simulink model "l1_ver113"  (Simulink 7.9)
settings: Solver=FixedStepDiscrete  SolverMode=SingleTasking  StartTime=0.0  StopTime=2048  RelTol=1e-3
undefined symbols (not defined in the file): sfp
libraries: xbsIndex_r4 x2413, casper_library_downconverter x256, xps_library x32, dspsigops x32, casper_library_misc x27, casper_library_flow_control x16, simulink x7
(part 5 of 24)

== system l1_ver113/dec_fir3 ==
  sync_in : Inport
  real1 : Inport  Port=2
  imag1 : Inport  Port=3
  real2 : Inport  Port=4
  imag2 : Inport  Port=5
  real3 : Inport  Port=6
  imag3 : Inport  Port=7
  real4 : Inport  Port=8
  imag4 : Inport  Port=9
  real5 : Inport  Port=10
  imag5 : Inport  Port=11
  real6 : Inport  Port=12
  imag6 : Inport  Port=13
  real7 : Inport  Port=14
  imag7 : Inport  Port=15
  real8 : Inport  Port=16
  imag8 : Inport  Port=17
  real9 : Inport  Port=18
  imag9 : Inport  Port=19
  real10 : Inport  Port=20
  imag10 : Inport  Port=21
  real11 : Inport  Port=22
  imag11 : Inport  Port=23
  real12 : Inport  Port=24
  imag12 : Inport  Port=25
  real13 : Inport  Port=26
  imag13 : Inport  Port=27
  real14 : Inport  Port=28
  imag14 : Inport  Port=29
  real15 : Inport  Port=30
  imag15 : Inport  Port=31
  real16 : Inport  Port=32
  imag16 : Inport  Port=33
  convert1 : xbsIndex_r4/Convert  gui_display_data_type=Fixed-point  arith_type="Signed  (2's comp)"  n_bits=32  bin_pt=31  float_type=Single  exp_bits=8  fraction_bits=24  quantization="Round  (unbiased: +/- Inf)"  overflow=Wrap  en=off  latency=2  dbl_ovrd=off  pipeline=off  block_type=convert
  convert2 : xbsIndex_r4/Convert  gui_display_data_type=Fixed-point  arith_type="Signed  (2's comp)"  n_bits=32  bin_pt=31  float_type=Single  exp_bits=8  fraction_bits=24  quantization="Round  (unbiased: +/- Inf)"  overflow=Wrap  en=off  latency=2  dbl_ovrd=off  pipeline=off  block_type=convert
  delay : xbsIndex_r4/Delay  rst=off  infoeditControl=Selection of Reset will increase slice count due to use of real FFs and instead of SRLs  en=off  latency=9  dbl_ovrd=off  reg_retiming=off  block_type=delay
  fir_dbl_col1 : Subsystem  AncestorBlock=casper_library_downconverter/fir_dbl_col  mask(fir_dbl_col){n_inputs=16, coeff=getfield( getfield( get_param( gcb,'UserData' ), 'parameters'),'coeff'), add_latency=1, mult_latency=2, coeff_bit_width=25, coeff_bin_pt=24, first_stage_hdl=on, adder_imp=DSP48}
      mask prompts: n_inputs "Inputs"; coeff "Coefficients"; add_latency "Add latency"; mult_latency "Mult Latency"; coeff_bit_width "Coefficient bit width"; coeff_bin_pt "Coefficient binary point"; first_stage_hdl "Implement first stage of adder trees on output as behavioural HDL"; adder_imp "Adder implementation"
      mask init: same as l1_ver113/dec_fir1/fir_dbl_col1
      mask Help: eval('xlWeb(''http://casper.berkeley.edu/wiki/Fir_dbl_col'')')
      mask StyleString: edit,edit,edit,edit,edit,edit,checkbox,popup(Behavioral|Fabric|DSP48)
  fir_dbl_col2 : Subsystem  AncestorBlock=casper_library_downconverter/fir_dbl_col  mask(fir_dbl_col){n_inputs=16, coeff=getfield( getfield( get_param( gcb,'UserData' ), 'parameters'),'coeff'), add_latency=1, mult_latency=2, coeff_bit_width=25, coeff_bin_pt=24, first_stage_hdl=on, adder_imp=DSP48}
      mask prompts: n_inputs "Inputs"; coeff "Coefficients"; add_latency "Add latency"; mult_latency "Mult Latency"; coeff_bit_width "Coefficient bit width"; coeff_bin_pt "Coefficient binary point"; first_stage_hdl "Implement first stage of adder trees on output as behavioural HDL"; adder_imp "Adder implementation"
      mask init: same as l1_ver113/dec_fir1/fir_dbl_col1
      mask Help: eval('xlWeb(''http://casper.berkeley.edu/wiki/Fir_dbl_col'')')
      mask StyleString: edit,edit,edit,edit,edit,edit,checkbox,popup(Behavioral|Fabric|DSP48)
  fir_dbl_col3 : Subsystem  AncestorBlock=casper_library_downconverter/fir_dbl_col  mask(fir_dbl_col){n_inputs=16, coeff=getfield( getfield( get_param( gcb,'UserData' ), 'parameters'),'coeff'), add_latency=1, mult_latency=2, coeff_bit_width=25, coeff_bin_pt=24, first_stage_hdl=on, adder_imp=DSP48}
      mask prompts: n_inputs "Inputs"; coeff "Coefficients"; add_latency "Add latency"; mult_latency "Mult Latency"; coeff_bit_width "Coefficient bit width"; coeff_bin_pt "Coefficient binary point"; first_stage_hdl "Implement first stage of adder trees on output as behavioural HDL"; adder_imp "Adder implementation"
      mask init: same as l1_ver113/dec_fir1/fir_dbl_col1
      mask Help: eval('xlWeb(''http://casper.berkeley.edu/wiki/Fir_dbl_col'')')
      mask StyleString: edit,edit,edit,edit,edit,edit,checkbox,popup(Behavioral|Fabric|DSP48)
  fir_dbl_col4 : Subsystem  AncestorBlock=casper_library_downconverter/fir_dbl_col  mask(fir_dbl_col){n_inputs=16, coeff=getfield( getfield( get_param( gcb,'UserData' ), 'parameters'),'coeff'), add_latency=1, mult_latency=2, coeff_bit_width=25, coeff_bin_pt=24, first_stage_hdl=on, adder_imp=DSP48}
      mask prompts: n_inputs "Inputs"; coeff "Coefficients"; add_latency "Add latency"; mult_latency "Mult Latency"; coeff_bit_width "Coefficient bit width"; coeff_bin_pt "Coefficient binary point"; first_stage_hdl "Implement first stage of adder trees on output as behavioural HDL"; adder_imp "Adder implementation"
      mask init: same as l1_ver113/dec_fir1/fir_dbl_col1
      mask Help: eval('xlWeb(''http://casper.berkeley.edu/wiki/Fir_dbl_col'')')
      mask StyleString: edit,edit,edit,edit,edit,edit,checkbox,popup(Behavioral|Fabric|DSP48)
  fir_dbl_col5 : Subsystem  AncestorBlock=casper_library_downconverter/fir_dbl_col  mask(fir_dbl_col){n_inputs=16, coeff=getfield( getfield( get_param( gcb,'UserData' ), 'parameters'),'coeff'), add_latency=1, mult_latency=2, coeff_bit_width=25, coeff_bin_pt=24, first_stage_hdl=on, adder_imp=DSP48}
      mask prompts: n_inputs "Inputs"; coeff "Coefficients"; add_latency "Add latency"; mult_latency "Mult Latency"; coeff_bit_width "Coefficient bit width"; coeff_bin_pt "Coefficient binary point"; first_stage_hdl "Implement first stage of adder trees on output as behavioural HDL"; adder_imp "Adder implementation"
      mask init: same as l1_ver113/dec_fir1/fir_dbl_col1
      mask Help: eval('xlWeb(''http://casper.berkeley.edu/wiki/Fir_dbl_col'')')
      mask StyleString: edit,edit,edit,edit,edit,edit,checkbox,popup(Behavioral|Fabric|DSP48)
  fir_dbl_col6 : Subsystem  AncestorBlock=casper_library_downconverter/fir_dbl_col  mask(fir_dbl_col){n_inputs=16, coeff=getfield( getfield( get_param( gcb,'UserData' ), 'parameters'),'coeff'), add_latency=1, mult_latency=2, coeff_bit_width=25, coeff_bin_pt=24, first_stage_hdl=on, adder_imp=DSP48}
      mask prompts: n_inputs "Inputs"; coeff "Coefficients"; add_latency "Add latency"; mult_latency "Mult Latency"; coeff_bit_width "Coefficient bit width"; coeff_bin_pt "Coefficient binary point"; first_stage_hdl "Implement first stage of adder trees on output as behavioural HDL"; adder_imp "Adder implementation"
      mask init: same as l1_ver113/dec_fir1/fir_dbl_col1
      mask Help: eval('xlWeb(''http://casper.berkeley.edu/wiki/Fir_dbl_col'')')
      mask StyleString: edit,edit,edit,edit,edit,edit,checkbox,popup(Behavioral|Fabric|DSP48)
  fir_dbl_col7 : Subsystem  AncestorBlock=casper_library_downconverter/fir_dbl_col  mask(fir_dbl_col){n_inputs=16, coeff=getfield( getfield( get_param( gcb,'UserData' ), 'parameters'),'coeff'), add_latency=1, mult_latency=2, coeff_bit_width=25, coeff_bin_pt=24, first_stage_hdl=on, adder_imp=DSP48}
      mask prompts: n_inputs "Inputs"; coeff "Coefficients"; add_latency "Add latency"; mult_latency "Mult Latency"; coeff_bit_width "Coefficient bit width"; coeff_bin_pt "Coefficient binary point"; first_stage_hdl "Implement first stage of adder trees on output as behavioural HDL"; adder_imp "Adder implementation"
      mask init: same as l1_ver113/dec_fir1/fir_dbl_col1
      mask Help: eval('xlWeb(''http://casper.berkeley.edu/wiki/Fir_dbl_col'')')
      mask StyleString: edit,edit,edit,edit,edit,edit,checkbox,popup(Behavioral|Fabric|DSP48)
  fir_dbl_col8 : Subsystem  AncestorBlock=casper_library_downconverter/fir_dbl_col  mask(fir_dbl_col){n_inputs=16, coeff=getfield( getfield( get_param( gcb,'UserData' ), 'parameters'),'coeff'), add_latency=1, mult_latency=2, coeff_bit_width=25, coeff_bin_pt=24, first_stage_hdl=on, adder_imp=DSP48}
      mask prompts: n_inputs "Inputs"; coeff "Coefficients"; add_latency "Add latency"; mult_latency "Mult Latency"; coeff_bit_width "Coefficient bit width"; coeff_bin_pt "Coefficient binary point"; first_stage_hdl "Implement first stage of adder trees on output as behavioural HDL"; adder_imp "Adder implementation"
      mask init: same as l1_ver113/dec_fir1/fir_dbl_col1
      mask Help: eval('xlWeb(''http://casper.berkeley.edu/wiki/Fir_dbl_col'')')
      mask StyleString: edit,edit,edit,edit,edit,edit,checkbox,popup(Behavioral|Fabric|DSP48)
  imag_sum : Subsystem  AncestorBlock=casper_library_misc/adder_tree  mask(adder_tree){n_inputs=8, latency=1, adder_imp=DSP48, first_stage_hdl=off}
      mask prompts: n_inputs "Number of Inputs"; latency "Add Latency"; adder_imp "Adder implementation"; first_stage_hdl "Use behavioural HDL for first stage of adders"
      mask help:
      | Sums all inputs using a tree of adds and delays.
      |
      | Number of inputs: The number of inputs to the block to be summed
      | Add latency: The number of clock cycles to perform one addition (affects timing)..
      | Adder implementation: choice of fabric or DSP48E core, or behavioral HDL
      | Use behavioural HDL for first stage of adders: Causes the first stage of adders to be absorbed into DSP blocks if multipliers precede them (Virtex 5). Overrides Adder implementation
      mask init:
      | adder_tree_init(gcb, ...
      |     'n_inputs', n_inputs, ...
      |     'latency', latency, ...
      |     'adder_imp', adder_imp, ...
      |     'first_stage_hdl', first_stage_hdl);
      settings: AttributesFormatString=latency 3
      mask Help: eval('xlWeb(''http://casper.berkeley.edu/wiki/Adder_tree'')')
      mask StyleString: edit,edit,popup(Behavioral|Fabric|DSP48),checkbox
  real_sum : Subsystem  AncestorBlock=casper_library_misc/adder_tree  mask(adder_tree){n_inputs=8, latency=1, adder_imp=DSP48, first_stage_hdl=off}
      mask prompts: n_inputs "Number of Inputs"; latency "Add Latency"; adder_imp "Adder implementation"; first_stage_hdl "Use behavioural HDL for first stage of adders"
      mask help:
      | Sums all inputs using a tree of adds and delays.
      |
      | Number of inputs: The number of inputs to the block to be summed
      | Add latency: The number of clock cycles to perform one addition (affects timing)..
      | Adder implementation: choice of fabric or DSP48E core, or behavioral HDL
      | Use behavioural HDL for first stage of adders: Causes the first stage of adders to be absorbed into DSP blocks if multipliers precede them (Virtex 5). Overrides Adder implementation
      mask init:
      | adder_tree_init(gcb, ...
      |     'n_inputs', n_inputs, ...
      |     'latency', latency, ...
      |     'adder_imp', adder_imp, ...
      |     'first_stage_hdl', first_stage_hdl);
      settings: AttributesFormatString=latency 3
      mask Help: eval('xlWeb(''http://casper.berkeley.edu/wiki/Adder_tree'')')
      mask StyleString: edit,edit,popup(Behavioral|Fabric|DSP48),checkbox
  ri_to_c : casper_library_misc/ri_to_c
  shift1 : xbsIndex_r4/Shift  shift_dir=Left  shift_bits=1  en=off  latency=0  precision=Full  arith_type="Signed  (2's comp)"  n_bits=16  bin_pt=14  quantization=Truncate  overflow=Wrap  dbl_ovrd=off  block_type=shift
  shift2 : xbsIndex_r4/Shift  shift_dir=Left  shift_bits=1  en=off  latency=0  precision=Full  arith_type="Signed  (2's comp)"  n_bits=16  bin_pt=14  quantization=Truncate  overflow=Wrap  dbl_ovrd=off  block_type=shift
  sync_out : Outport
  dout : Outport  Port=2
  wires:
  real1/1 -> fir_dbl_col1/1(real1)
  imag1/1 -> fir_dbl_col1/2(imag1)
  real2/1 -> fir_dbl_col1/3(real2)
  imag2/1 -> fir_dbl_col1/4(imag2)
  real3/1 -> fir_dbl_col1/5(real3)
  imag3/1 -> fir_dbl_col1/6(imag3)
  real4/1 -> fir_dbl_col1/7(real4)
  imag4/1 -> fir_dbl_col1/8(imag4)
  real5/1 -> fir_dbl_col1/9(real5)
  imag5/1 -> fir_dbl_col1/10(imag5)
  real6/1 -> fir_dbl_col1/11(real6)
  imag6/1 -> fir_dbl_col1/12(imag6)
  real7/1 -> fir_dbl_col1/13(real7)
  imag7/1 -> fir_dbl_col1/14(imag7)
  real8/1 -> fir_dbl_col1/15(real8)
  imag8/1 -> fir_dbl_col1/16(imag8)
  real9/1 -> fir_dbl_col1/17(real9)
  imag9/1 -> fir_dbl_col1/18(imag9)
  real10/1 -> fir_dbl_col1/19(real10)
  imag10/1 -> fir_dbl_col1/20(imag10)
  real11/1 -> fir_dbl_col1/21(real11)
  imag11/1 -> fir_dbl_col1/22(imag11)
  real12/1 -> fir_dbl_col1/23(real12)
  imag12/1 -> fir_dbl_col1/24(imag12)
  real13/1 -> fir_dbl_col1/25(real13)
  imag13/1 -> fir_dbl_col1/26(imag13)
  real14/1 -> fir_dbl_col1/27(real14)
  imag14/1 -> fir_dbl_col1/28(imag14)
  real15/1 -> fir_dbl_col1/29(real15)
  imag15/1 -> fir_dbl_col1/30(imag15)
  real16/1 -> fir_dbl_col1/31(real16)
  imag16/1 -> fir_dbl_col1/32(imag16)
  fir_dbl_col1/65(real_sum) -> real_sum/2(din1)
  fir_dbl_col1/66(imag_sum) -> imag_sum/2(din1)
  fir_dbl_col1/1(real_out1) -> fir_dbl_col2/1(real1)
  fir_dbl_col1/2(imag_out1) -> fir_dbl_col2/2(imag1)
  fir_dbl_col1/3(real_out2) -> fir_dbl_col2/3(real2)
  fir_dbl_col1/4(imag_out2) -> fir_dbl_col2/4(imag2)
  fir_dbl_col1/5(real_out3) -> fir_dbl_col2/5(real3)
  fir_dbl_col1/6(imag_out3) -> fir_dbl_col2/6(imag3)
  fir_dbl_col1/7(real_out4) -> fir_dbl_col2/7(real4)
  fir_dbl_col1/8(imag_out4) -> fir_dbl_col2/8(imag4)
  fir_dbl_col1/9(real_out5) -> fir_dbl_col2/9(real5)
  fir_dbl_col1/10(imag_out5) -> fir_dbl_col2/10(imag5)
  fir_dbl_col1/11(real_out6) -> fir_dbl_col2/11(real6)
  fir_dbl_col1/12(imag_out6) -> fir_dbl_col2/12(imag6)
  fir_dbl_col1/13(real_out7) -> fir_dbl_col2/13(real7)
  fir_dbl_col1/14(imag_out7) -> fir_dbl_col2/14(imag7)
  fir_dbl_col1/15(real_out8) -> fir_dbl_col2/15(real8)
  fir_dbl_col1/16(imag_out8) -> fir_dbl_col2/16(imag8)
  fir_dbl_col1/17(real_out9) -> fir_dbl_col2/17(real9)
  fir_dbl_col1/18(imag_out9) -> fir_dbl_col2/18(imag9)
  fir_dbl_col1/19(real_out10) -> fir_dbl_col2/19(real10)
  fir_dbl_col1/20(imag_out10) -> fir_dbl_col2/20(imag10)
  fir_dbl_col1/21(real_out11) -> fir_dbl_col2/21(real11)
  fir_dbl_col1/22(imag_out11) -> fir_dbl_col2/22(imag11)
  fir_dbl_col1/23(real_out12) -> fir_dbl_col2/23(real12)
  fir_dbl_col1/24(imag_out12) -> fir_dbl_col2/24(imag12)
  fir_dbl_col1/25(real_out13) -> fir_dbl_col2/25(real13)
  fir_dbl_col1/26(imag_out13) -> fir_dbl_col2/26(imag13)
  fir_dbl_col1/27(real_out14) -> fir_dbl_col2/27(real14)
  fir_dbl_col1/28(imag_out14) -> fir_dbl_col2/28(imag14)
  fir_dbl_col1/29(real_out15) -> fir_dbl_col2/29(real15)
  fir_dbl_col1/30(imag_out15) -> fir_dbl_col2/30(imag15)
  fir_dbl_col1/31(real_out16) -> fir_dbl_col2/31(real16)
  fir_dbl_col1/32(imag_out16) -> fir_dbl_col2/32(imag16)
  fir_dbl_col2/65(real_sum) -> real_sum/3(din2)
  fir_dbl_col2/66(imag_sum) -> imag_sum/3(din2)
  fir_dbl_col2/1(real_out1) -> fir_dbl_col3/1(real1)
  fir_dbl_col2/2(imag_out1) -> fir_dbl_col3/2(imag1)
  fir_dbl_col2/3(real_out2) -> fir_dbl_col3/3(real2)
  fir_dbl_col2/4(imag_out2) -> fir_dbl_col3/4(imag2)
  fir_dbl_col2/5(real_out3) -> fir_dbl_col3/5(real3)
  fir_dbl_col2/6(imag_out3) -> fir_dbl_col3/6(imag3)
  fir_dbl_col2/7(real_out4) -> fir_dbl_col3/7(real4)
  fir_dbl_col2/8(imag_out4) -> fir_dbl_col3/8(imag4)
  fir_dbl_col2/9(real_out5) -> fir_dbl_col3/9(real5)
  fir_dbl_col2/10(imag_out5) -> fir_dbl_col3/10(imag5)
  fir_dbl_col2/11(real_out6) -> fir_dbl_col3/11(real6)
  fir_dbl_col2/12(imag_out6) -> fir_dbl_col3/12(imag6)
  fir_dbl_col2/13(real_out7) -> fir_dbl_col3/13(real7)
  fir_dbl_col2/14(imag_out7) -> fir_dbl_col3/14(imag7)
  fir_dbl_col2/15(real_out8) -> fir_dbl_col3/15(real8)
  fir_dbl_col2/16(imag_out8) -> fir_dbl_col3/16(imag8)
  fir_dbl_col2/17(real_out9) -> fir_dbl_col3/17(real9)
  fir_dbl_col2/18(imag_out9) -> fir_dbl_col3/18(imag9)
  fir_dbl_col2/19(real_out10) -> fir_dbl_col3/19(real10)
  fir_dbl_col2/20(imag_out10) -> fir_dbl_col3/20(imag10)
  fir_dbl_col2/21(real_out11) -> fir_dbl_col3/21(real11)
  fir_dbl_col2/22(imag_out11) -> fir_dbl_col3/22(imag11)
  fir_dbl_col2/23(real_out12) -> fir_dbl_col3/23(real12)
  fir_dbl_col2/24(imag_out12) -> fir_dbl_col3/24(imag12)
  fir_dbl_col2/25(real_out13) -> fir_dbl_col3/25(real13)
  fir_dbl_col2/26(imag_out13) -> fir_dbl_col3/26(imag13)
  fir_dbl_col2/27(real_out14) -> fir_dbl_col3/27(real14)
  fir_dbl_col2/28(imag_out14) -> fir_dbl_col3/28(imag14)
  fir_dbl_col2/29(real_out15) -> fir_dbl_col3/29(real15)
  fir_dbl_col2/30(imag_out15) -> fir_dbl_col3/30(imag15)
  fir_dbl_col2/31(real_out16) -> fir_dbl_col3/31(real16)
  fir_dbl_col2/32(imag_out16) -> fir_dbl_col3/32(imag16)
  fir_dbl_col3/65(real_sum) -> real_sum/4(din3)
  fir_dbl_col3/66(imag_sum) -> imag_sum/4(din3)
  fir_dbl_col3/1(real_out1) -> fir_dbl_col4/1(real1)
  fir_dbl_col3/2(imag_out1) -> fir_dbl_col4/2(imag1)
  fir_dbl_col3/3(real_out2) -> fir_dbl_col4/3(real2)
  fir_dbl_col3/4(imag_out2) -> fir_dbl_col4/4(imag2)
  fir_dbl_col3/5(real_out3) -> fir_dbl_col4/5(real3)
  fir_dbl_col3/6(imag_out3) -> fir_dbl_col4/6(imag3)
  fir_dbl_col3/7(real_out4) -> fir_dbl_col4/7(real4)
  fir_dbl_col3/8(imag_out4) -> fir_dbl_col4/8(imag4)
  fir_dbl_col3/9(real_out5) -> fir_dbl_col4/9(real5)
  fir_dbl_col3/10(imag_out5) -> fir_dbl_col4/10(imag5)
  fir_dbl_col3/11(real_out6) -> fir_dbl_col4/11(real6)
  fir_dbl_col3/12(imag_out6) -> fir_dbl_col4/12(imag6)
  fir_dbl_col3/13(real_out7) -> fir_dbl_col4/13(real7)
  fir_dbl_col3/14(imag_out7) -> fir_dbl_col4/14(imag7)
  fir_dbl_col3/15(real_out8) -> fir_dbl_col4/15(real8)
  fir_dbl_col3/16(imag_out8) -> fir_dbl_col4/16(imag8)
  fir_dbl_col3/17(real_out9) -> fir_dbl_col4/17(real9)
  fir_dbl_col3/18(imag_out9) -> fir_dbl_col4/18(imag9)
  fir_dbl_col3/19(real_out10) -> fir_dbl_col4/19(real10)
  fir_dbl_col3/20(imag_out10) -> fir_dbl_col4/20(imag10)
  fir_dbl_col3/21(real_out11) -> fir_dbl_col4/21(real11)
  fir_dbl_col3/22(imag_out11) -> fir_dbl_col4/22(imag11)
  fir_dbl_col3/23(real_out12) -> fir_dbl_col4/23(real12)
  fir_dbl_col3/24(imag_out12) -> fir_dbl_col4/24(imag12)
  fir_dbl_col3/25(real_out13) -> fir_dbl_col4/25(real13)
  fir_dbl_col3/26(imag_out13) -> fir_dbl_col4/26(imag13)
  fir_dbl_col3/27(real_out14) -> fir_dbl_col4/27(real14)
  fir_dbl_col3/28(imag_out14) -> fir_dbl_col4/28(imag14)
  fir_dbl_col3/29(real_out15) -> fir_dbl_col4/29(real15)
  fir_dbl_col3/30(imag_out15) -> fir_dbl_col4/30(imag15)
  fir_dbl_col3/31(real_out16) -> fir_dbl_col4/31(real16)
  fir_dbl_col3/32(imag_out16) -> fir_dbl_col4/32(imag16)
  fir_dbl_col4/65(real_sum) -> real_sum/5(din4)
  fir_dbl_col4/66(imag_sum) -> imag_sum/5(din4)
  fir_dbl_col4/1(real_out1) -> fir_dbl_col5/1(real1)
  fir_dbl_col4/2(imag_out1) -> fir_dbl_col5/2(imag1)
  fir_dbl_col4/3(real_out2) -> fir_dbl_col5/3(real2)
  fir_dbl_col4/4(imag_out2) -> fir_dbl_col5/4(imag2)
  fir_dbl_col4/5(real_out3) -> fir_dbl_col5/5(real3)
  fir_dbl_col4/6(imag_out3) -> fir_dbl_col5/6(imag3)
  fir_dbl_col4/7(real_out4) -> fir_dbl_col5/7(real4)
  fir_dbl_col4/8(imag_out4) -> fir_dbl_col5/8(imag4)
  fir_dbl_col4/9(real_out5) -> fir_dbl_col5/9(real5)
  fir_dbl_col4/10(imag_out5) -> fir_dbl_col5/10(imag5)
  fir_dbl_col4/11(real_out6) -> fir_dbl_col5/11(real6)
  fir_dbl_col4/12(imag_out6) -> fir_dbl_col5/12(imag6)
  fir_dbl_col4/13(real_out7) -> fir_dbl_col5/13(real7)
  fir_dbl_col4/14(imag_out7) -> fir_dbl_col5/14(imag7)
  fir_dbl_col4/15(real_out8) -> fir_dbl_col5/15(real8)
  fir_dbl_col4/16(imag_out8) -> fir_dbl_col5/16(imag8)
  fir_dbl_col4/17(real_out9) -> fir_dbl_col5/17(real9)
  fir_dbl_col4/18(imag_out9) -> fir_dbl_col5/18(imag9)
  fir_dbl_col4/19(real_out10) -> fir_dbl_col5/19(real10)
  fir_dbl_col4/20(imag_out10) -> fir_dbl_col5/20(imag10)
  fir_dbl_col4/21(real_out11) -> fir_dbl_col5/21(real11)
  fir_dbl_col4/22(imag_out11) -> fir_dbl_col5/22(imag11)
  fir_dbl_col4/23(real_out12) -> fir_dbl_col5/23(real12)
  fir_dbl_col4/24(imag_out12) -> fir_dbl_col5/24(imag12)
  fir_dbl_col4/25(real_out13) -> fir_dbl_col5/25(real13)
  fir_dbl_col4/26(imag_out13) -> fir_dbl_col5/26(imag13)
  fir_dbl_col4/27(real_out14) -> fir_dbl_col5/27(real14)
  fir_dbl_col4/28(imag_out14) -> fir_dbl_col5/28(imag14)
  fir_dbl_col4/29(real_out15) -> fir_dbl_col5/29(real15)
  fir_dbl_col4/30(imag_out15) -> fir_dbl_col5/30(imag15)
  fir_dbl_col4/31(real_out16) -> fir_dbl_col5/31(real16)
  fir_dbl_col4/32(imag_out16) -> fir_dbl_col5/32(imag16)
  fir_dbl_col5/65(real_sum) -> real_sum/6(din5)
  fir_dbl_col5/66(imag_sum) -> imag_sum/6(din5)
  fir_dbl_col5/1(real_out1) -> fir_dbl_col6/1(real1)
  fir_dbl_col5/2(imag_out1) -> fir_dbl_col6/2(imag1)
  fir_dbl_col5/3(real_out2) -> fir_dbl_col6/3(real2)
  fir_dbl_col5/4(imag_out2) -> fir_dbl_col6/4(imag2)
  fir_dbl_col5/5(real_out3) -> fir_dbl_col6/5(real3)
  fir_dbl_col5/6(imag_out3) -> fir_dbl_col6/6(imag3)
  fir_dbl_col5/7(real_out4) -> fir_dbl_col6/7(real4)
  fir_dbl_col5/8(imag_out4) -> fir_dbl_col6/8(imag4)
  fir_dbl_col5/9(real_out5) -> fir_dbl_col6/9(real5)
  fir_dbl_col5/10(imag_out5) -> fir_dbl_col6/10(imag5)
  fir_dbl_col5/11(real_out6) -> fir_dbl_col6/11(real6)
  fir_dbl_col5/12(imag_out6) -> fir_dbl_col6/12(imag6)
  fir_dbl_col5/13(real_out7) -> fir_dbl_col6/13(real7)
  fir_dbl_col5/14(imag_out7) -> fir_dbl_col6/14(imag7)
  fir_dbl_col5/15(real_out8) -> fir_dbl_col6/15(real8)
  fir_dbl_col5/16(imag_out8) -> fir_dbl_col6/16(imag8)
  fir_dbl_col5/17(real_out9) -> fir_dbl_col6/17(real9)
  fir_dbl_col5/18(imag_out9) -> fir_dbl_col6/18(imag9)
  fir_dbl_col5/19(real_out10) -> fir_dbl_col6/19(real10)
  fir_dbl_col5/20(imag_out10) -> fir_dbl_col6/20(imag10)
  fir_dbl_col5/21(real_out11) -> fir_dbl_col6/21(real11)
  fir_dbl_col5/22(imag_out11) -> fir_dbl_col6/22(imag11)
  fir_dbl_col5/23(real_out12) -> fir_dbl_col6/23(real12)
  fir_dbl_col5/24(imag_out12) -> fir_dbl_col6/24(imag12)
  fir_dbl_col5/25(real_out13) -> fir_dbl_col6/25(real13)
  fir_dbl_col5/26(imag_out13) -> fir_dbl_col6/26(imag13)
  fir_dbl_col5/27(real_out14) -> fir_dbl_col6/27(real14)
  fir_dbl_col5/28(imag_out14) -> fir_dbl_col6/28(imag14)
  fir_dbl_col5/29(real_out15) -> fir_dbl_col6/29(real15)
  fir_dbl_col5/30(imag_out15) -> fir_dbl_col6/30(imag15)
  fir_dbl_col5/31(real_out16) -> fir_dbl_col6/31(real16)
  fir_dbl_col5/32(imag_out16) -> fir_dbl_col6/32(imag16)
  fir_dbl_col6/65(real_sum) -> real_sum/7(din6)
  fir_dbl_col6/66(imag_sum) -> imag_sum/7(din6)
  fir_dbl_col6/1(real_out1) -> fir_dbl_col7/1(real1)
  fir_dbl_col6/2(imag_out1) -> fir_dbl_col7/2(imag1)
  fir_dbl_col6/3(real_out2) -> fir_dbl_col7/3(real2)
  fir_dbl_col6/4(imag_out2) -> fir_dbl_col7/4(imag2)
  fir_dbl_col6/5(real_out3) -> fir_dbl_col7/5(real3)
  fir_dbl_col6/6(imag_out3) -> fir_dbl_col7/6(imag3)
  fir_dbl_col6/7(real_out4) -> fir_dbl_col7/7(real4)
  fir_dbl_col6/8(imag_out4) -> fir_dbl_col7/8(imag4)
  fir_dbl_col6/9(real_out5) -> fir_dbl_col7/9(real5)
  fir_dbl_col6/10(imag_out5) -> fir_dbl_col7/10(imag5)
  fir_dbl_col6/11(real_out6) -> fir_dbl_col7/11(real6)
  fir_dbl_col6/12(imag_out6) -> fir_dbl_col7/12(imag6)
  fir_dbl_col6/13(real_out7) -> fir_dbl_col7/13(real7)
  fir_dbl_col6/14(imag_out7) -> fir_dbl_col7/14(imag7)
  fir_dbl_col6/15(real_out8) -> fir_dbl_col7/15(real8)
  fir_dbl_col6/16(imag_out8) -> fir_dbl_col7/16(imag8)
  fir_dbl_col6/17(real_out9) -> fir_dbl_col7/17(real9)
  fir_dbl_col6/18(imag_out9) -> fir_dbl_col7/18(imag9)
  fir_dbl_col6/19(real_out10) -> fir_dbl_col7/19(real10)
  fir_dbl_col6/20(imag_out10) -> fir_dbl_col7/20(imag10)
  fir_dbl_col6/21(real_out11) -> fir_dbl_col7/21(real11)
  fir_dbl_col6/22(imag_out11) -> fir_dbl_col7/22(imag11)
  fir_dbl_col6/23(real_out12) -> fir_dbl_col7/23(real12)
  fir_dbl_col6/24(imag_out12) -> fir_dbl_col7/24(imag12)
  fir_dbl_col6/25(real_out13) -> fir_dbl_col7/25(real13)
  fir_dbl_col6/26(imag_out13) -> fir_dbl_col7/26(imag13)
  fir_dbl_col6/27(real_out14) -> fir_dbl_col7/27(real14)
  fir_dbl_col6/28(imag_out14) -> fir_dbl_col7/28(imag14)
  fir_dbl_col6/29(real_out15) -> fir_dbl_col7/29(real15)
  fir_dbl_col6/30(imag_out15) -> fir_dbl_col7/30(imag15)
  fir_dbl_col6/31(real_out16) -> fir_dbl_col7/31(real16)
  fir_dbl_col6/32(imag_out16) -> fir_dbl_col7/32(imag16)
  fir_dbl_col7/65(real_sum) -> real_sum/8(din7)
  fir_dbl_col7/66(imag_sum) -> imag_sum/8(din7)
  fir_dbl_col7/1(real_out1) -> fir_dbl_col8/1(real1)
  fir_dbl_col7/2(imag_out1) -> fir_dbl_col8/2(imag1)
  fir_dbl_col7/3(real_out2) -> fir_dbl_col8/3(real2)
  fir_dbl_col7/4(imag_out2) -> fir_dbl_col8/4(imag2)
  fir_dbl_col7/5(real_out3) -> fir_dbl_col8/5(real3)
  fir_dbl_col7/6(imag_out3) -> fir_dbl_col8/6(imag3)
  fir_dbl_col7/7(real_out4) -> fir_dbl_col8/7(real4)
  fir_dbl_col7/8(imag_out4) -> fir_dbl_col8/8(imag4)
  fir_dbl_col7/9(real_out5) -> fir_dbl_col8/9(real5)
  fir_dbl_col7/10(imag_out5) -> fir_dbl_col8/10(imag5)
  fir_dbl_col7/11(real_out6) -> fir_dbl_col8/11(real6)
  fir_dbl_col7/12(imag_out6) -> fir_dbl_col8/12(imag6)
  fir_dbl_col7/13(real_out7) -> fir_dbl_col8/13(real7)
  fir_dbl_col7/14(imag_out7) -> fir_dbl_col8/14(imag7)
  fir_dbl_col7/15(real_out8) -> fir_dbl_col8/15(real8)
  fir_dbl_col7/16(imag_out8) -> fir_dbl_col8/16(imag8)
  fir_dbl_col7/17(real_out9) -> fir_dbl_col8/17(real9)
  fir_dbl_col7/18(imag_out9) -> fir_dbl_col8/18(imag9)
  fir_dbl_col7/19(real_out10) -> fir_dbl_col8/19(real10)
  fir_dbl_col7/20(imag_out10) -> fir_dbl_col8/20(imag10)
  fir_dbl_col7/21(real_out11) -> fir_dbl_col8/21(real11)
  fir_dbl_col7/22(imag_out11) -> fir_dbl_col8/22(imag11)
  fir_dbl_col7/23(real_out12) -> fir_dbl_col8/23(real12)
  fir_dbl_col7/24(imag_out12) -> fir_dbl_col8/24(imag12)
  fir_dbl_col7/25(real_out13) -> fir_dbl_col8/25(real13)
  fir_dbl_col7/26(imag_out13) -> fir_dbl_col8/26(imag13)
  fir_dbl_col7/27(real_out14) -> fir_dbl_col8/27(real14)
  fir_dbl_col7/28(imag_out14) -> fir_dbl_col8/28(imag14)
  fir_dbl_col7/29(real_out15) -> fir_dbl_col8/29(real15)
  fir_dbl_col7/30(imag_out15) -> fir_dbl_col8/30(imag15)
  fir_dbl_col7/31(real_out16) -> fir_dbl_col8/31(real16)
  fir_dbl_col7/32(imag_out16) -> fir_dbl_col8/32(imag16)
  fir_dbl_col8/65(real_sum) -> real_sum/9(din8)
  fir_dbl_col8/66(imag_sum) -> imag_sum/9(din8)
  real_sum/2(dout) -> shift1/1
  imag_sum/2(dout) -> shift2/1
  shift1/1 -> convert1/1
  shift2/1 -> convert2/1
  convert1/1 -> ri_to_c/1
  convert2/1 -> ri_to_c/2
  ri_to_c/1 -> dout/1
  sync_in/1 -> delay/1
  delay/1 -> real_sum/1(sync), imag_sum/1(sync)
  real_sum/1(sync_out) -> sync_out/1
  fir_dbl_col2/33(real_back_out1) -> fir_dbl_col1/33(real_back1)
  fir_dbl_col2/34(imag_back_out1) -> fir_dbl_col1/34(imag_back1)
  fir_dbl_col2/35(real_back_out2) -> fir_dbl_col1/35(real_back2)
  fir_dbl_col2/36(imag_back_out2) -> fir_dbl_col1/36(imag_back2)
  fir_dbl_col2/37(real_back_out3) -> fir_dbl_col1/37(real_back3)
  fir_dbl_col2/38(imag_back_out3) -> fir_dbl_col1/38(imag_back3)
  fir_dbl_col2/39(real_back_out4) -> fir_dbl_col1/39(real_back4)
  fir_dbl_col2/40(imag_back_out4) -> fir_dbl_col1/40(imag_back4)
  fir_dbl_col2/41(real_back_out5) -> fir_dbl_col1/41(real_back5)
  fir_dbl_col2/42(imag_back_out5) -> fir_dbl_col1/42(imag_back5)
  fir_dbl_col2/43(real_back_out6) -> fir_dbl_col1/43(real_back6)
  fir_dbl_col2/44(imag_back_out6) -> fir_dbl_col1/44(imag_back6)
  fir_dbl_col2/45(real_back_out7) -> fir_dbl_col1/45(real_back7)
  fir_dbl_col2/46(imag_back_out7) -> fir_dbl_col1/46(imag_back7)
  fir_dbl_col2/47(real_back_out8) -> fir_dbl_col1/47(real_back8)
  fir_dbl_col2/48(imag_back_out8) -> fir_dbl_col1/48(imag_back8)
  fir_dbl_col2/49(real_back_out9) -> fir_dbl_col1/49(real_back9)
  fir_dbl_col2/50(imag_back_out9) -> fir_dbl_col1/50(imag_back9)
  fir_dbl_col2/51(real_back_out10) -> fir_dbl_col1/51(real_back10)
  fir_dbl_col2/52(imag_back_out10) -> fir_dbl_col1/52(imag_back10)
  fir_dbl_col2/53(real_back_out11) -> fir_dbl_col1/53(real_back11)
  fir_dbl_col2/54(imag_back_out11) -> fir_dbl_col1/54(imag_back11)
  fir_dbl_col2/55(real_back_out12) -> fir_dbl_col1/55(real_back12)
  fir_dbl_col2/56(imag_back_out12) -> fir_dbl_col1/56(imag_back12)
  fir_dbl_col2/57(real_back_out13) -> fir_dbl_col1/57(real_back13)
  fir_dbl_col2/58(imag_back_out13) -> fir_dbl_col1/58(imag_back13)
  fir_dbl_col2/59(real_back_out14) -> fir_dbl_col1/59(real_back14)
  fir_dbl_col2/60(imag_back_out14) -> fir_dbl_col1/60(imag_back14)
  fir_dbl_col2/61(real_back_out15) -> fir_dbl_col1/61(real_back15)
  fir_dbl_col2/62(imag_back_out15) -> fir_dbl_col1/62(imag_back15)
  fir_dbl_col2/63(real_back_out16) -> fir_dbl_col1/63(real_back16)
  fir_dbl_col2/64(imag_back_out16) -> fir_dbl_col1/64(imag_back16)
  fir_dbl_col3/33(real_back_out1) -> fir_dbl_col2/33(real_back1)
  fir_dbl_col3/34(imag_back_out1) -> fir_dbl_col2/34(imag_back1)
  fir_dbl_col3/35(real_back_out2) -> fir_dbl_col2/35(real_back2)
  fir_dbl_col3/36(imag_back_out2) -> fir_dbl_col2/36(imag_back2)
  fir_dbl_col3/37(real_back_out3) -> fir_dbl_col2/37(real_back3)
  fir_dbl_col3/38(imag_back_out3) -> fir_dbl_col2/38(imag_back3)
  fir_dbl_col3/39(real_back_out4) -> fir_dbl_col2/39(real_back4)
  fir_dbl_col3/40(imag_back_out4) -> fir_dbl_col2/40(imag_back4)
  fir_dbl_col3/41(real_back_out5) -> fir_dbl_col2/41(real_back5)
  fir_dbl_col3/42(imag_back_out5) -> fir_dbl_col2/42(imag_back5)
  fir_dbl_col3/43(real_back_out6) -> fir_dbl_col2/43(real_back6)
  fir_dbl_col3/44(imag_back_out6) -> fir_dbl_col2/44(imag_back6)
  fir_dbl_col3/45(real_back_out7) -> fir_dbl_col2/45(real_back7)
  fir_dbl_col3/46(imag_back_out7) -> fir_dbl_col2/46(imag_back7)
  fir_dbl_col3/47(real_back_out8) -> fir_dbl_col2/47(real_back8)
  fir_dbl_col3/48(imag_back_out8) -> fir_dbl_col2/48(imag_back8)
  fir_dbl_col3/49(real_back_out9) -> fir_dbl_col2/49(real_back9)
  fir_dbl_col3/50(imag_back_out9) -> fir_dbl_col2/50(imag_back9)
  fir_dbl_col3/51(real_back_out10) -> fir_dbl_col2/51(real_back10)
  fir_dbl_col3/52(imag_back_out10) -> fir_dbl_col2/52(imag_back10)
  fir_dbl_col3/53(real_back_out11) -> fir_dbl_col2/53(real_back11)
  fir_dbl_col3/54(imag_back_out11) -> fir_dbl_col2/54(imag_back11)
  fir_dbl_col3/55(real_back_out12) -> fir_dbl_col2/55(real_back12)
  fir_dbl_col3/56(imag_back_out12) -> fir_dbl_col2/56(imag_back12)
  fir_dbl_col3/57(real_back_out13) -> fir_dbl_col2/57(real_back13)
  fir_dbl_col3/58(imag_back_out13) -> fir_dbl_col2/58(imag_back13)
  fir_dbl_col3/59(real_back_out14) -> fir_dbl_col2/59(real_back14)
  fir_dbl_col3/60(imag_back_out14) -> fir_dbl_col2/60(imag_back14)
  fir_dbl_col3/61(real_back_out15) -> fir_dbl_col2/61(real_back15)
  fir_dbl_col3/62(imag_back_out15) -> fir_dbl_col2/62(imag_back15)
  fir_dbl_col3/63(real_back_out16) -> fir_dbl_col2/63(real_back16)
  fir_dbl_col3/64(imag_back_out16) -> fir_dbl_col2/64(imag_back16)
  fir_dbl_col4/33(real_back_out1) -> fir_dbl_col3/33(real_back1)
  fir_dbl_col4/34(imag_back_out1) -> fir_dbl_col3/34(imag_back1)
  fir_dbl_col4/35(real_back_out2) -> fir_dbl_col3/35(real_back2)
  fir_dbl_col4/36(imag_back_out2) -> fir_dbl_col3/36(imag_back2)
  fir_dbl_col4/37(real_back_out3) -> fir_dbl_col3/37(real_back3)
  fir_dbl_col4/38(imag_back_out3) -> fir_dbl_col3/38(imag_back3)
  fir_dbl_col4/39(real_back_out4) -> fir_dbl_col3/39(real_back4)
  fir_dbl_col4/40(imag_back_out4) -> fir_dbl_col3/40(imag_back4)
  fir_dbl_col4/41(real_back_out5) -> fir_dbl_col3/41(real_back5)
  fir_dbl_col4/42(imag_back_out5) -> fir_dbl_col3/42(imag_back5)
  fir_dbl_col4/43(real_back_out6) -> fir_dbl_col3/43(real_back6)
  fir_dbl_col4/44(imag_back_out6) -> fir_dbl_col3/44(imag_back6)
  fir_dbl_col4/45(real_back_out7) -> fir_dbl_col3/45(real_back7)
  fir_dbl_col4/46(imag_back_out7) -> fir_dbl_col3/46(imag_back7)
  fir_dbl_col4/47(real_back_out8) -> fir_dbl_col3/47(real_back8)
  fir_dbl_col4/48(imag_back_out8) -> fir_dbl_col3/48(imag_back8)
  fir_dbl_col4/49(real_back_out9) -> fir_dbl_col3/49(real_back9)
  fir_dbl_col4/50(imag_back_out9) -> fir_dbl_col3/50(imag_back9)
  fir_dbl_col4/51(real_back_out10) -> fir_dbl_col3/51(real_back10)
  fir_dbl_col4/52(imag_back_out10) -> fir_dbl_col3/52(imag_back10)
  fir_dbl_col4/53(real_back_out11) -> fir_dbl_col3/53(real_back11)
  fir_dbl_col4/54(imag_back_out11) -> fir_dbl_col3/54(imag_back11)
  fir_dbl_col4/55(real_back_out12) -> fir_dbl_col3/55(real_back12)
  fir_dbl_col4/56(imag_back_out12) -> fir_dbl_col3/56(imag_back12)
  fir_dbl_col4/57(real_back_out13) -> fir_dbl_col3/57(real_back13)
  fir_dbl_col4/58(imag_back_out13) -> fir_dbl_col3/58(imag_back13)
  fir_dbl_col4/59(real_back_out14) -> fir_dbl_col3/59(real_back14)
  fir_dbl_col4/60(imag_back_out14) -> fir_dbl_col3/60(imag_back14)
  fir_dbl_col4/61(real_back_out15) -> fir_dbl_col3/61(real_back15)
  fir_dbl_col4/62(imag_back_out15) -> fir_dbl_col3/62(imag_back15)
  fir_dbl_col4/63(real_back_out16) -> fir_dbl_col3/63(real_back16)
  fir_dbl_col4/64(imag_back_out16) -> fir_dbl_col3/64(imag_back16)
  fir_dbl_col5/33(real_back_out1) -> fir_dbl_col4/33(real_back1)
  fir_dbl_col5/34(imag_back_out1) -> fir_dbl_col4/34(imag_back1)
  fir_dbl_col5/35(real_back_out2) -> fir_dbl_col4/35(real_back2)
  fir_dbl_col5/36(imag_back_out2) -> fir_dbl_col4/36(imag_back2)
  fir_dbl_col5/37(real_back_out3) -> fir_dbl_col4/37(real_back3)
  fir_dbl_col5/38(imag_back_out3) -> fir_dbl_col4/38(imag_back3)
  fir_dbl_col5/39(real_back_out4) -> fir_dbl_col4/39(real_back4)
  fir_dbl_col5/40(imag_back_out4) -> fir_dbl_col4/40(imag_back4)
  fir_dbl_col5/41(real_back_out5) -> fir_dbl_col4/41(real_back5)
  fir_dbl_col5/42(imag_back_out5) -> fir_dbl_col4/42(imag_back5)
  fir_dbl_col5/43(real_back_out6) -> fir_dbl_col4/43(real_back6)
  fir_dbl_col5/44(imag_back_out6) -> fir_dbl_col4/44(imag_back6)
  fir_dbl_col5/45(real_back_out7) -> fir_dbl_col4/45(real_back7)
  fir_dbl_col5/46(imag_back_out7) -> fir_dbl_col4/46(imag_back7)
  fir_dbl_col5/47(real_back_out8) -> fir_dbl_col4/47(real_back8)
  fir_dbl_col5/48(imag_back_out8) -> fir_dbl_col4/48(imag_back8)
  fir_dbl_col5/49(real_back_out9) -> fir_dbl_col4/49(real_back9)
  fir_dbl_col5/50(imag_back_out9) -> fir_dbl_col4/50(imag_back9)
  fir_dbl_col5/51(real_back_out10) -> fir_dbl_col4/51(real_back10)
  fir_dbl_col5/52(imag_back_out10) -> fir_dbl_col4/52(imag_back10)
  fir_dbl_col5/53(real_back_out11) -> fir_dbl_col4/53(real_back11)
  fir_dbl_col5/54(imag_back_out11) -> fir_dbl_col4/54(imag_back11)
  fir_dbl_col5/55(real_back_out12) -> fir_dbl_col4/55(real_back12)
  fir_dbl_col5/56(imag_back_out12) -> fir_dbl_col4/56(imag_back12)
  fir_dbl_col5/57(real_back_out13) -> fir_dbl_col4/57(real_back13)
  fir_dbl_col5/58(imag_back_out13) -> fir_dbl_col4/58(imag_back13)
  fir_dbl_col5/59(real_back_out14) -> fir_dbl_col4/59(real_back14)
  fir_dbl_col5/60(imag_back_out14) -> fir_dbl_col4/60(imag_back14)
  fir_dbl_col5/61(real_back_out15) -> fir_dbl_col4/61(real_back15)
  fir_dbl_col5/62(imag_back_out15) -> fir_dbl_col4/62(imag_back15)
  fir_dbl_col5/63(real_back_out16) -> fir_dbl_col4/63(real_back16)
  fir_dbl_col5/64(imag_back_out16) -> fir_dbl_col4/64(imag_back16)
  fir_dbl_col6/33(real_back_out1) -> fir_dbl_col5/33(real_back1)
  fir_dbl_col6/34(imag_back_out1) -> fir_dbl_col5/34(imag_back1)
  fir_dbl_col6/35(real_back_out2) -> fir_dbl_col5/35(real_back2)
  fir_dbl_col6/36(imag_back_out2) -> fir_dbl_col5/36(imag_back2)
  fir_dbl_col6/37(real_back_out3) -> fir_dbl_col5/37(real_back3)
  fir_dbl_col6/38(imag_back_out3) -> fir_dbl_col5/38(imag_back3)
  fir_dbl_col6/39(real_back_out4) -> fir_dbl_col5/39(real_back4)
  fir_dbl_col6/40(imag_back_out4) -> fir_dbl_col5/40(imag_back4)
  fir_dbl_col6/41(real_back_out5) -> fir_dbl_col5/41(real_back5)
  fir_dbl_col6/42(imag_back_out5) -> fir_dbl_col5/42(imag_back5)
  fir_dbl_col6/43(real_back_out6) -> fir_dbl_col5/43(real_back6)
  fir_dbl_col6/44(imag_back_out6) -> fir_dbl_col5/44(imag_back6)
  fir_dbl_col6/45(real_back_out7) -> fir_dbl_col5/45(real_back7)
  fir_dbl_col6/46(imag_back_out7) -> fir_dbl_col5/46(imag_back7)
  fir_dbl_col6/47(real_back_out8) -> fir_dbl_col5/47(real_back8)
  fir_dbl_col6/48(imag_back_out8) -> fir_dbl_col5/48(imag_back8)
  fir_dbl_col6/49(real_back_out9) -> fir_dbl_col5/49(real_back9)
  fir_dbl_col6/50(imag_back_out9) -> fir_dbl_col5/50(imag_back9)
  fir_dbl_col6/51(real_back_out10) -> fir_dbl_col5/51(real_back10)
  fir_dbl_col6/52(imag_back_out10) -> fir_dbl_col5/52(imag_back10)
  fir_dbl_col6/53(real_back_out11) -> fir_dbl_col5/53(real_back11)
  fir_dbl_col6/54(imag_back_out11) -> fir_dbl_col5/54(imag_back11)
  fir_dbl_col6/55(real_back_out12) -> fir_dbl_col5/55(real_back12)
  fir_dbl_col6/56(imag_back_out12) -> fir_dbl_col5/56(imag_back12)
  fir_dbl_col6/57(real_back_out13) -> fir_dbl_col5/57(real_back13)
  fir_dbl_col6/58(imag_back_out13) -> fir_dbl_col5/58(imag_back13)
  fir_dbl_col6/59(real_back_out14) -> fir_dbl_col5/59(real_back14)
  fir_dbl_col6/60(imag_back_out14) -> fir_dbl_col5/60(imag_back14)
  fir_dbl_col6/61(real_back_out15) -> fir_dbl_col5/61(real_back15)
  fir_dbl_col6/62(imag_back_out15) -> fir_dbl_col5/62(imag_back15)
  fir_dbl_col6/63(real_back_out16) -> fir_dbl_col5/63(real_back16)
  fir_dbl_col6/64(imag_back_out16) -> fir_dbl_col5/64(imag_back16)
  fir_dbl_col7/33(real_back_out1) -> fir_dbl_col6/33(real_back1)
  fir_dbl_col7/34(imag_back_out1) -> fir_dbl_col6/34(imag_back1)
  fir_dbl_col7/35(real_back_out2) -> fir_dbl_col6/35(real_back2)
  fir_dbl_col7/36(imag_back_out2) -> fir_dbl_col6/36(imag_back2)
  fir_dbl_col7/37(real_back_out3) -> fir_dbl_col6/37(real_back3)
  fir_dbl_col7/38(imag_back_out3) -> fir_dbl_col6/38(imag_back3)
  fir_dbl_col7/39(real_back_out4) -> fir_dbl_col6/39(real_back4)
  fir_dbl_col7/40(imag_back_out4) -> fir_dbl_col6/40(imag_back4)
  fir_dbl_col7/41(real_back_out5) -> fir_dbl_col6/41(real_back5)
  fir_dbl_col7/42(imag_back_out5) -> fir_dbl_col6/42(imag_back5)
  fir_dbl_col7/43(real_back_out6) -> fir_dbl_col6/43(real_back6)
  fir_dbl_col7/44(imag_back_out6) -> fir_dbl_col6/44(imag_back6)
  fir_dbl_col7/45(real_back_out7) -> fir_dbl_col6/45(real_back7)
  fir_dbl_col7/46(imag_back_out7) -> fir_dbl_col6/46(imag_back7)
  fir_dbl_col7/47(real_back_out8) -> fir_dbl_col6/47(real_back8)
  fir_dbl_col7/48(imag_back_out8) -> fir_dbl_col6/48(imag_back8)
  fir_dbl_col7/49(real_back_out9) -> fir_dbl_col6/49(real_back9)
  fir_dbl_col7/50(imag_back_out9) -> fir_dbl_col6/50(imag_back9)
  fir_dbl_col7/51(real_back_out10) -> fir_dbl_col6/51(real_back10)
  fir_dbl_col7/52(imag_back_out10) -> fir_dbl_col6/52(imag_back10)
  fir_dbl_col7/53(real_back_out11) -> fir_dbl_col6/53(real_back11)
  fir_dbl_col7/54(imag_back_out11) -> fir_dbl_col6/54(imag_back11)
  fir_dbl_col7/55(real_back_out12) -> fir_dbl_col6/55(real_back12)
  fir_dbl_col7/56(imag_back_out12) -> fir_dbl_col6/56(imag_back12)
  fir_dbl_col7/57(real_back_out13) -> fir_dbl_col6/57(real_back13)
  fir_dbl_col7/58(imag_back_out13) -> fir_dbl_col6/58(imag_back13)
  fir_dbl_col7/59(real_back_out14) -> fir_dbl_col6/59(real_back14)
  fir_dbl_col7/60(imag_back_out14) -> fir_dbl_col6/60(imag_back14)
  fir_dbl_col7/61(real_back_out15) -> fir_dbl_col6/61(real_back15)
  fir_dbl_col7/62(imag_back_out15) -> fir_dbl_col6/62(imag_back15)
  fir_dbl_col7/63(real_back_out16) -> fir_dbl_col6/63(real_back16)
  fir_dbl_col7/64(imag_back_out16) -> fir_dbl_col6/64(imag_back16)
  fir_dbl_col8/33(real_back_out1) -> fir_dbl_col7/33(real_back1)
  fir_dbl_col8/34(imag_back_out1) -> fir_dbl_col7/34(imag_back1)
  fir_dbl_col8/35(real_back_out2) -> fir_dbl_col7/35(real_back2)
  fir_dbl_col8/36(imag_back_out2) -> fir_dbl_col7/36(imag_back2)
  fir_dbl_col8/37(real_back_out3) -> fir_dbl_col7/37(real_back3)
  fir_dbl_col8/38(imag_back_out3) -> fir_dbl_col7/38(imag_back3)
  fir_dbl_col8/39(real_back_out4) -> fir_dbl_col7/39(real_back4)
  fir_dbl_col8/40(imag_back_out4) -> fir_dbl_col7/40(imag_back4)
  fir_dbl_col8/41(real_back_out5) -> fir_dbl_col7/41(real_back5)
  fir_dbl_col8/42(imag_back_out5) -> fir_dbl_col7/42(imag_back5)
  fir_dbl_col8/43(real_back_out6) -> fir_dbl_col7/43(real_back6)
  fir_dbl_col8/44(imag_back_out6) -> fir_dbl_col7/44(imag_back6)
  fir_dbl_col8/45(real_back_out7) -> fir_dbl_col7/45(real_back7)
  fir_dbl_col8/46(imag_back_out7) -> fir_dbl_col7/46(imag_back7)
  fir_dbl_col8/47(real_back_out8) -> fir_dbl_col7/47(real_back8)
  fir_dbl_col8/48(imag_back_out8) -> fir_dbl_col7/48(imag_back8)
  fir_dbl_col8/49(real_back_out9) -> fir_dbl_col7/49(real_back9)
  fir_dbl_col8/50(imag_back_out9) -> fir_dbl_col7/50(imag_back9)
  fir_dbl_col8/51(real_back_out10) -> fir_dbl_col7/51(real_back10)
  fir_dbl_col8/52(imag_back_out10) -> fir_dbl_col7/52(imag_back10)
  fir_dbl_col8/53(real_back_out11) -> fir_dbl_col7/53(real_back11)
  fir_dbl_col8/54(imag_back_out11) -> fir_dbl_col7/54(imag_back11)
  fir_dbl_col8/55(real_back_out12) -> fir_dbl_col7/55(real_back12)
  fir_dbl_col8/56(imag_back_out12) -> fir_dbl_col7/56(imag_back12)
  fir_dbl_col8/57(real_back_out13) -> fir_dbl_col7/57(real_back13)
  fir_dbl_col8/58(imag_back_out13) -> fir_dbl_col7/58(imag_back13)
  fir_dbl_col8/59(real_back_out14) -> fir_dbl_col7/59(real_back14)
  fir_dbl_col8/60(imag_back_out14) -> fir_dbl_col7/60(imag_back14)
  fir_dbl_col8/61(real_back_out15) -> fir_dbl_col7/61(real_back15)
  fir_dbl_col8/62(imag_back_out15) -> fir_dbl_col7/62(imag_back15)
  fir_dbl_col8/63(real_back_out16) -> fir_dbl_col7/63(real_back16)
  fir_dbl_col8/64(imag_back_out16) -> fir_dbl_col7/64(imag_back16)
  fir_dbl_col8/1(real_out1) -> fir_dbl_col8/33(real_back1)
  fir_dbl_col8/2(imag_out1) -> fir_dbl_col8/34(imag_back1)
  fir_dbl_col8/3(real_out2) -> fir_dbl_col8/35(real_back2)
  fir_dbl_col8/4(imag_out2) -> fir_dbl_col8/36(imag_back2)
  fir_dbl_col8/5(real_out3) -> fir_dbl_col8/37(real_back3)
  fir_dbl_col8/6(imag_out3) -> fir_dbl_col8/38(imag_back3)
  fir_dbl_col8/7(real_out4) -> fir_dbl_col8/39(real_back4)
  fir_dbl_col8/8(imag_out4) -> fir_dbl_col8/40(imag_back4)
  fir_dbl_col8/9(real_out5) -> fir_dbl_col8/41(real_back5)
  fir_dbl_col8/10(imag_out5) -> fir_dbl_col8/42(imag_back5)
  fir_dbl_col8/11(real_out6) -> fir_dbl_col8/43(real_back6)
  fir_dbl_col8/12(imag_out6) -> fir_dbl_col8/44(imag_back6)
  fir_dbl_col8/13(real_out7) -> fir_dbl_col8/45(real_back7)
  fir_dbl_col8/14(imag_out7) -> fir_dbl_col8/46(imag_back7)
  fir_dbl_col8/15(real_out8) -> fir_dbl_col8/47(real_back8)
  fir_dbl_col8/16(imag_out8) -> fir_dbl_col8/48(imag_back8)
  fir_dbl_col8/17(real_out9) -> fir_dbl_col8/49(real_back9)
  fir_dbl_col8/18(imag_out9) -> fir_dbl_col8/50(imag_back9)
  fir_dbl_col8/19(real_out10) -> fir_dbl_col8/51(real_back10)
  fir_dbl_col8/20(imag_out10) -> fir_dbl_col8/52(imag_back10)
  fir_dbl_col8/21(real_out11) -> fir_dbl_col8/53(real_back11)
  fir_dbl_col8/22(imag_out11) -> fir_dbl_col8/54(imag_back11)
  fir_dbl_col8/23(real_out12) -> fir_dbl_col8/55(real_back12)
  fir_dbl_col8/24(imag_out12) -> fir_dbl_col8/56(imag_back12)
  fir_dbl_col8/25(real_out13) -> fir_dbl_col8/57(real_back13)
  fir_dbl_col8/26(imag_out13) -> fir_dbl_col8/58(imag_back13)
  fir_dbl_col8/27(real_out14) -> fir_dbl_col8/59(real_back14)
  fir_dbl_col8/28(imag_out14) -> fir_dbl_col8/60(imag_back14)
  fir_dbl_col8/29(real_out15) -> fir_dbl_col8/61(real_back15)
  fir_dbl_col8/30(imag_out15) -> fir_dbl_col8/62(imag_back15)
  fir_dbl_col8/31(real_out16) -> fir_dbl_col8/63(real_back16)
  fir_dbl_col8/32(imag_out16) -> fir_dbl_col8/64(imag_back16)

== system l1_ver113/downshiftbyeight ==
  In1 : Inport
  In2 : Inport  Port=2
  In3 : Inport  Port=3
  In4 : Inport  Port=4
  In5 : Inport  Port=5
  In6 : Inport  Port=6
  In7 : Inport  Port=7
  In8 : Inport  Port=8
  In9 : Inport  Port=9
  In10 : Inport  Port=10
  In11 : Inport  Port=11
  In12 : Inport  Port=12
  In13 : Inport  Port=13
  In14 : Inport  Port=14
  In15 : Inport  Port=15
  In16 : Inport  Port=16
  In17 : Inport  Port=17
  In18 : Inport  Port=18
  In19 : Inport  Port=19
  In20 : Inport  Port=20
  In21 : Inport  Port=21
  In22 : Inport  Port=22
  In23 : Inport  Port=23
  In24 : Inport  Port=24
  In25 : Inport  Port=25
  In26 : Inport  Port=26
  In27 : Inport  Port=27
  In28 : Inport  Port=28
  In29 : Inport  Port=29
  In30 : Inport  Port=30
  In31 : Inport  Port=31
  In32 : Inport  Port=32
  Reinterpret{,1..31} (32 alike) : xbsIndex_r4/Reinterpret  force_arith_type=off  arith_type=Unsigned  force_bin_pt=on  bin_pt=11  block_type=reinterpret
  Shift{,1..31} (32 alike) : xbsIndex_r4/Shift  shift_dir=Right  shift_bits=3  en=off  latency=0  precision=User Defined  arith_type="Signed  (2's comp)"  n_bits=12  bin_pt=10  quantization=Truncate  overflow=Wrap  dbl_ovrd=off  block_type=shift
  Out1 : Outport
  Out2 : Outport  Port=2
  Out3 : Outport  Port=3
  Out4 : Outport  Port=4
  Out5 : Outport  Port=5
  Out6 : Outport  Port=6
  Out7 : Outport  Port=7
  Out8 : Outport  Port=8
  Out9 : Outport  Port=9
  Out10 : Outport  Port=10
  Out11 : Outport  Port=11
  Out12 : Outport  Port=12
  Out13 : Outport  Port=13
  Out14 : Outport  Port=14
  Out15 : Outport  Port=15
  Out16 : Outport  Port=16
  Out17 : Outport  Port=17
  Out18 : Outport  Port=18
  Out19 : Outport  Port=19
  Out20 : Outport  Port=20
  Out21 : Outport  Port=21
  Out22 : Outport  Port=22
  Out23 : Outport  Port=23
  Out24 : Outport  Port=24
  Out25 : Outport  Port=25
  Out26 : Outport  Port=26
  Out27 : Outport  Port=27
  Out28 : Outport  Port=28
  Out29 : Outport  Port=29
  Out30 : Outport  Port=30
  Out31 : Outport  Port=31
  Out32 : Outport  Port=32
  wires:
  Shift/1 -> Reinterpret/1
  In1/1 -> Shift/1
  Reinterpret/1 -> Out1/1
  Shift1/1 -> Reinterpret1/1
  In2/1 -> Shift1/1
  Reinterpret1/1 -> Out2/1
  Shift2/1 -> Reinterpret2/1
  In3/1 -> Shift2/1
  Reinterpret2/1 -> Out3/1
  Shift3/1 -> Reinterpret3/1
  In4/1 -> Shift3/1
  Reinterpret3/1 -> Out4/1
  Shift4/1 -> Reinterpret4/1
  In5/1 -> Shift4/1
  Reinterpret4/1 -> Out5/1
  Shift5/1 -> Reinterpret5/1
  In6/1 -> Shift5/1
  Reinterpret5/1 -> Out6/1
  Shift6/1 -> Reinterpret6/1
  In7/1 -> Shift6/1
  Reinterpret6/1 -> Out7/1
  Shift7/1 -> Reinterpret7/1
  In8/1 -> Shift7/1
  Reinterpret7/1 -> Out8/1
  Shift8/1 -> Reinterpret8/1
  In9/1 -> Shift8/1
  Reinterpret8/1 -> Out9/1
  Shift9/1 -> Reinterpret9/1
  In10/1 -> Shift9/1
  Reinterpret9/1 -> Out10/1
  Shift10/1 -> Reinterpret10/1
  In11/1 -> Shift10/1
  Reinterpret10/1 -> Out11/1
  Shift11/1 -> Reinterpret11/1
  In12/1 -> Shift11/1
  Reinterpret11/1 -> Out12/1
  Shift12/1 -> Reinterpret12/1
  In13/1 -> Shift12/1
  Reinterpret12/1 -> Out13/1
  Shift13/1 -> Reinterpret13/1
  In14/1 -> Shift13/1
  Reinterpret13/1 -> Out14/1
  Shift14/1 -> Reinterpret14/1
  In15/1 -> Shift14/1
  Reinterpret14/1 -> Out15/1
  Shift15/1 -> Reinterpret15/1
  In16/1 -> Shift15/1
  Reinterpret15/1 -> Out16/1
  Shift16/1 -> Reinterpret16/1
  In17/1 -> Shift16/1
  Reinterpret16/1 -> Out17/1
  Shift17/1 -> Reinterpret17/1
  In18/1 -> Shift17/1
  Reinterpret17/1 -> Out18/1
  Shift24/1 -> Reinterpret24/1
  In19/1 -> Shift24/1
  Reinterpret24/1 -> Out19/1
  Shift25/1 -> Reinterpret25/1
  In20/1 -> Shift25/1
  Reinterpret25/1 -> Out20/1
  Shift26/1 -> Reinterpret26/1
  In21/1 -> Shift26/1
  Reinterpret26/1 -> Out21/1
  Shift27/1 -> Reinterpret27/1
  In22/1 -> Shift27/1
  Reinterpret27/1 -> Out22/1
  Shift28/1 -> Reinterpret28/1
  In23/1 -> Shift28/1
  Reinterpret28/1 -> Out23/1
  Shift29/1 -> Reinterpret29/1
  In24/1 -> Shift29/1
  Reinterpret29/1 -> Out24/1
  Shift30/1 -> Reinterpret30/1
  In25/1 -> Shift30/1
  Reinterpret30/1 -> Out25/1
  Shift31/1 -> Reinterpret31/1
  In26/1 -> Shift31/1
  Reinterpret31/1 -> Out26/1
  Shift18/1 -> Reinterpret18/1
  In27/1 -> Shift18/1
  Reinterpret18/1 -> Out27/1
  Shift19/1 -> Reinterpret19/1
  In28/1 -> Shift19/1
  Reinterpret19/1 -> Out28/1
  Shift20/1 -> Reinterpret20/1
  In29/1 -> Shift20/1
  Reinterpret20/1 -> Out29/1
  Shift21/1 -> Reinterpret21/1
  In30/1 -> Shift21/1
  Reinterpret21/1 -> Out30/1
  Shift22/1 -> Reinterpret22/1
  In31/1 -> Shift22/1
  Reinterpret22/1 -> Out31/1
  Shift23/1 -> Reinterpret23/1
  In32/1 -> Shift23/1
  Reinterpret23/1 -> Out32/1
